simulink model "roach2_fengine"  (Simulink 8.1)
settings: Solver=VariableStepDiscrete  SolverMode=SingleTasking  StartTime=0.0  StopTime=10.0  RelTol=1e-3
undefined symbols (not defined in the file or in its folder): sfp
libraries: xbsIndex_r4 x3838, xps_library x64, casper_library_sources x16, casper_library_misc x13, casper_library_delays x7, casper_library_reorder x3
(part 4 of 43)

== system roach2_fengine/eq/7 ==
  note: Counter has length nchan=number of channels of the fft.
      | BRAM has length nchan as well. Every input/channel
      | has its own eq value.
      | Top half of the BRAM, has coefficients for first input,
      | second half for the rest.
  note: shared brams have a latency of three
  in : Inport
  sync : Inport  Port=2
  Constant4 : xbsIndex_r4/Constant  const=0  gui_display_data_type=Fixed-point  arith_type=Unsigned  n_bits=18  bin_pt=0  preci_type=Single  exp_width=8  frac_width=24  explicit_period=on  period=1  equ=P=C  opselect=C  inp2=PCIN>>17  opr=+  inp1=P  carry=CIN  dbl_ovrd=off  block_type=constant
  Constant5 : xbsIndex_r4/Constant  const=0  gui_display_data_type=Fixed-point  arith_type=Boolean  n_bits=1  bin_pt=0  preci_type=Single  exp_width=8  frac_width=24  explicit_period=on  period=1  equ=P=C  opselect=C  inp2=PCIN>>17  opr=+  inp1=P  carry=CIN  dbl_ovrd=off  block_type=constant
  Counter : xbsIndex_r4/Counter  cnt_type=Free Running  cnt_to=Inf  operation=Up  start_count=0  cnt_by_val=1  arith_type=Unsigned  n_bits=nchan  bin_pt=0  load_pin=off  rst=on  en=off  explicit_period=off  period=1  dbl_ovrd=off  use_behavioral_HDL=off  implementation=Fabric  use_rpm=on  block_type=counter
      settings: xl_area=[0, 0, 0, 0, 0, 0, 0]
  Delay1 : xbsIndex_r4/Delay  rst=off  infoeditControl=Selection of Reset will increase slice count due to use of real FFs and instead of SRLs  en=off  latency=4  dbl_ovrd=off  reg_retiming=on  block_type=delay
      settings: xl_area=[0, 0, 0, 0, 0, 0, 0]
  Delay11 : xbsIndex_r4/Delay  rst=off  infoeditControl=Selection of Reset will increase slice count due to use of real FFs and instead of SRLs  en=off  latency=4  dbl_ovrd=off  reg_retiming=on  block_type=delay
      settings: xl_area=[0, 0, 0, 0, 0, 0, 0]
  Delay2 : xbsIndex_r4/Delay  rst=off  infoeditControl=Selection of Reset will increase slice count due to use of real FFs and instead of SRLs  en=off  latency=3  dbl_ovrd=off  reg_retiming=off  block_type=delay
      settings: xl_area=[0, 0, 0, 0, 0, 0, 0]
  Delay3 : xbsIndex_r4/Delay  rst=off  infoeditControl=Selection of Reset will increase slice count due to use of real FFs and instead of SRLs  en=off  latency=4  dbl_ovrd=off  reg_retiming=on  block_type=delay
      settings: xl_area=[0, 0, 0, 0, 0, 0, 0]
  Delay4 : xbsIndex_r4/Delay  rst=off  infoeditControl=Selection of Reset will increase slice count due to use of real FFs and instead of SRLs  en=off  latency=5  dbl_ovrd=off  reg_retiming=off  block_type=delay
      settings: xl_area=[0, 0, 0, 0, 0, 0, 0]
  Delay5 : xbsIndex_r4/Delay  rst=off  infoeditControl=Selection of Reset will increase slice count due to use of real FFs and instead of SRLs  en=off  latency=3  dbl_ovrd=off  reg_retiming=off  block_type=delay
      settings: xl_area=[0, 0, 0, 0, 0, 0, 0]
  Slice1 : xbsIndex_r4/Slice  nbits=18  boolean_output=off  mode=Lower Bit Location + Width  bit1=0  base1=MSB of Input  bit0=0  base0=LSB of Input  dbl_ovrd=off  block_type=slice
      settings: block_version=10.1.3
  coeffs : Subsystem  Tag=xps:bram  AncestorBlock=xps_library/Shared BRAM  mask{arith_type=Unsigned, addr_width=nchan, data_width=32, reg_prim_output=on, reg_core_output=on, optimization=Minimum_Area, data_bin_pt=0, init_vals=[0:2^10-1], sample_rate=1}
      mask prompts: arith_type "Output Data Type"; addr_width "Address width"; data_width "Data Width"; reg_prim_output "Register Primitive Output"; reg_core_output "Register Core Output"; data_bin_pt "Data Binary Point"; init_vals "Initial 32-bit values (simulation only)"; sample_rate "Sample rate"
      mask init:
      | shared_bram_mask;
      mask TypeOptions arith_type: Unsigned | Signed  (2's comp)
      mask TypeOptions data_width: 8 | 16 | 32 | 64 | 128
      mask TypeOptions optimization: Minimum_Area | Low_Power
  round : Subsystem
      settings: AttributesFormatString=latency=5
  dout : Outport
  sync_out : Outport  Port=2
  clip : Outport  Port=3
  wires:
  Constant4/1 -> coeffs/2(data_in)
  Constant5/1 -> coeffs/3(we)
  sync/1 -> Counter/1, Delay5/1
  round/1(dout) -> dout/1
  in/1 -> Delay2/1
  Delay4/1 -> sync_out/1
  round/2(clip) -> clip/1
  coeffs/1(data_out) -> Slice1/1
  Counter/1 -> coeffs/1(addr)
  Delay1/1 -> round/2(scale)
  Slice1/1 -> Delay1/1
  Delay11/1 -> round/1(din)
  Delay2/1 -> Delay11/1
  Delay3/1 -> Delay4/1
  Delay5/1 -> Delay3/1

== system roach2_fengine/eq/8 ==
  note: shared brams have a latency of three
  note: Counter has length nchan=number of channels of the fft.
      | BRAM has length nchan as well. Every input/channel
      | has its own eq value.
      | Top half of the BRAM, has coefficients for first input,
      | second half for the rest.
  in : Inport
  sync : Inport  Port=2
  Constant4 : xbsIndex_r4/Constant  const=0  gui_display_data_type=Fixed-point  arith_type=Unsigned  n_bits=18  bin_pt=0  preci_type=Single  exp_width=8  frac_width=24  explicit_period=on  period=1  equ=P=C  opselect=C  inp2=PCIN>>17  opr=+  inp1=P  carry=CIN  dbl_ovrd=off  block_type=constant
  Constant5 : xbsIndex_r4/Constant  const=0  gui_display_data_type=Fixed-point  arith_type=Boolean  n_bits=1  bin_pt=0  preci_type=Single  exp_width=8  frac_width=24  explicit_period=on  period=1  equ=P=C  opselect=C  inp2=PCIN>>17  opr=+  inp1=P  carry=CIN  dbl_ovrd=off  block_type=constant
  Counter : xbsIndex_r4/Counter  cnt_type=Free Running  cnt_to=Inf  operation=Up  start_count=0  cnt_by_val=1  arith_type=Unsigned  n_bits=nchan  bin_pt=0  load_pin=off  rst=on  en=off  explicit_period=off  period=1  dbl_ovrd=off  use_behavioral_HDL=off  implementation=Fabric  use_rpm=on  block_type=counter
      settings: xl_area=[0, 0, 0, 0, 0, 0, 0]
  Delay1 : xbsIndex_r4/Delay  rst=off  infoeditControl=Selection of Reset will increase slice count due to use of real FFs and instead of SRLs  en=off  latency=4  dbl_ovrd=off  reg_retiming=on  block_type=delay
      settings: xl_area=[0, 0, 0, 0, 0, 0, 0]
  Delay11 : xbsIndex_r4/Delay  rst=off  infoeditControl=Selection of Reset will increase slice count due to use of real FFs and instead of SRLs  en=off  latency=4  dbl_ovrd=off  reg_retiming=on  block_type=delay
      settings: xl_area=[0, 0, 0, 0, 0, 0, 0]
  Delay2 : xbsIndex_r4/Delay  rst=off  infoeditControl=Selection of Reset will increase slice count due to use of real FFs and instead of SRLs  en=off  latency=3  dbl_ovrd=off  reg_retiming=off  block_type=delay
      settings: xl_area=[0, 0, 0, 0, 0, 0, 0]
  Delay3 : xbsIndex_r4/Delay  rst=off  infoeditControl=Selection of Reset will increase slice count due to use of real FFs and instead of SRLs  en=off  latency=4  dbl_ovrd=off  reg_retiming=on  block_type=delay
      settings: xl_area=[0, 0, 0, 0, 0, 0, 0]
  Delay4 : xbsIndex_r4/Delay  rst=off  infoeditControl=Selection of Reset will increase slice count due to use of real FFs and instead of SRLs  en=off  latency=5  dbl_ovrd=off  reg_retiming=off  block_type=delay
      settings: xl_area=[0, 0, 0, 0, 0, 0, 0]
  Delay5 : xbsIndex_r4/Delay  rst=off  infoeditControl=Selection of Reset will increase slice count due to use of real FFs and instead of SRLs  en=off  latency=3  dbl_ovrd=off  reg_retiming=off  block_type=delay
      settings: xl_area=[0, 0, 0, 0, 0, 0, 0]
  Slice1 : xbsIndex_r4/Slice  nbits=18  boolean_output=off  mode=Lower Bit Location + Width  bit1=0  base1=MSB of Input  bit0=0  base0=LSB of Input  dbl_ovrd=off  block_type=slice
      settings: block_version=10.1.3
  coeffs : Subsystem  Tag=xps:bram  AncestorBlock=xps_library/Shared BRAM  mask{arith_type=Unsigned, addr_width=nchan, data_width=32, reg_prim_output=on, reg_core_output=on, optimization=Minimum_Area, data_bin_pt=0, init_vals=[0:2^10-1], sample_rate=1}
      mask prompts: arith_type "Output Data Type"; addr_width "Address width"; data_width "Data Width"; reg_prim_output "Register Primitive Output"; reg_core_output "Register Core Output"; data_bin_pt "Data Binary Point"; init_vals "Initial 32-bit values (simulation only)"; sample_rate "Sample rate"
      mask init:
      | shared_bram_mask;
      mask TypeOptions arith_type: Unsigned | Signed  (2's comp)
      mask TypeOptions data_width: 8 | 16 | 32 | 64 | 128
      mask TypeOptions optimization: Minimum_Area | Low_Power
  round : Subsystem
      settings: AttributesFormatString=latency=5
  dout : Outport
  sync_out : Outport  Port=2
  clip : Outport  Port=3
  wires:
  Delay5/1 -> Delay3/1
  Delay3/1 -> Delay4/1
  Delay2/1 -> Delay11/1
  Delay11/1 -> round/1(din)
  Slice1/1 -> Delay1/1
  Delay1/1 -> round/2(scale)
  Counter/1 -> coeffs/1(addr)
  coeffs/1(data_out) -> Slice1/1
  round/2(clip) -> clip/1
  Delay4/1 -> sync_out/1
  in/1 -> Delay2/1
  round/1(dout) -> dout/1
  sync/1 -> Delay5/1, Counter/1
  Constant5/1 -> coeffs/3(we)
  Constant4/1 -> coeffs/2(data_in)

== system roach2_fengine/eq/9 ==
  note: shared brams have a latency of three
  note: Counter has length nchan=number of channels of the fft.
      | BRAM has length nchan as well. Every input/channel
      | has its own eq value.
      | Top half of the BRAM, has coefficients for first input,
      | second half for the rest.
  in : Inport
  sync : Inport  Port=2
  Constant4 : xbsIndex_r4/Constant  const=0  gui_display_data_type=Fixed-point  arith_type=Unsigned  n_bits=18  bin_pt=0  preci_type=Single  exp_width=8  frac_width=24  explicit_period=on  period=1  equ=P=C  opselect=C  inp2=PCIN>>17  opr=+  inp1=P  carry=CIN  dbl_ovrd=off  block_type=constant
  Constant5 : xbsIndex_r4/Constant  const=0  gui_display_data_type=Fixed-point  arith_type=Boolean  n_bits=1  bin_pt=0  preci_type=Single  exp_width=8  frac_width=24  explicit_period=on  period=1  equ=P=C  opselect=C  inp2=PCIN>>17  opr=+  inp1=P  carry=CIN  dbl_ovrd=off  block_type=constant
  Counter : xbsIndex_r4/Counter  cnt_type=Free Running  cnt_to=Inf  operation=Up  start_count=0  cnt_by_val=1  arith_type=Unsigned  n_bits=nchan  bin_pt=0  load_pin=off  rst=on  en=off  explicit_period=off  period=1  dbl_ovrd=off  use_behavioral_HDL=off  implementation=Fabric  use_rpm=on  block_type=counter
      settings: xl_area=[0, 0, 0, 0, 0, 0, 0]
  Delay1 : xbsIndex_r4/Delay  rst=off  infoeditControl=Selection of Reset will increase slice count due to use of real FFs and instead of SRLs  en=off  latency=4  dbl_ovrd=off  reg_retiming=on  block_type=delay
      settings: xl_area=[0, 0, 0, 0, 0, 0, 0]
  Delay11 : xbsIndex_r4/Delay  rst=off  infoeditControl=Selection of Reset will increase slice count due to use of real FFs and instead of SRLs  en=off  latency=4  dbl_ovrd=off  reg_retiming=on  block_type=delay
      settings: xl_area=[0, 0, 0, 0, 0, 0, 0]
  Delay2 : xbsIndex_r4/Delay  rst=off  infoeditControl=Selection of Reset will increase slice count due to use of real FFs and instead of SRLs  en=off  latency=3  dbl_ovrd=off  reg_retiming=off  block_type=delay
      settings: xl_area=[0, 0, 0, 0, 0, 0, 0]
  Delay3 : xbsIndex_r4/Delay  rst=off  infoeditControl=Selection of Reset will increase slice count due to use of real FFs and instead of SRLs  en=off  latency=4  dbl_ovrd=off  reg_retiming=on  block_type=delay
      settings: xl_area=[0, 0, 0, 0, 0, 0, 0]
  Delay4 : xbsIndex_r4/Delay  rst=off  infoeditControl=Selection of Reset will increase slice count due to use of real FFs and instead of SRLs  en=off  latency=5  dbl_ovrd=off  reg_retiming=off  block_type=delay
      settings: xl_area=[0, 0, 0, 0, 0, 0, 0]
  Delay5 : xbsIndex_r4/Delay  rst=off  infoeditControl=Selection of Reset will increase slice count due to use of real FFs and instead of SRLs  en=off  latency=3  dbl_ovrd=off  reg_retiming=off  block_type=delay
      settings: xl_area=[0, 0, 0, 0, 0, 0, 0]
  Slice1 : xbsIndex_r4/Slice  nbits=18  boolean_output=off  mode=Lower Bit Location + Width  bit1=0  base1=MSB of Input  bit0=0  base0=LSB of Input  dbl_ovrd=off  block_type=slice
      settings: block_version=10.1.3
  coeffs : Subsystem  Tag=xps:bram  AncestorBlock=xps_library/Shared BRAM  mask{arith_type=Unsigned, addr_width=nchan, data_width=32, reg_prim_output=on, reg_core_output=on, optimization=Minimum_Area, data_bin_pt=0, init_vals=[0:2^10-1], sample_rate=1}
      mask prompts: arith_type "Output Data Type"; addr_width "Address width"; data_width "Data Width"; reg_prim_output "Register Primitive Output"; reg_core_output "Register Core Output"; data_bin_pt "Data Binary Point"; init_vals "Initial 32-bit values (simulation only)"; sample_rate "Sample rate"
      mask init:
      | shared_bram_mask;
      mask TypeOptions arith_type: Unsigned | Signed  (2's comp)
      mask TypeOptions data_width: 8 | 16 | 32 | 64 | 128
      mask TypeOptions optimization: Minimum_Area | Low_Power
  round : Subsystem
      settings: AttributesFormatString=latency=5
  dout : Outport
  sync_out : Outport  Port=2
  clip : Outport  Port=3
  wires:
  Delay5/1 -> Delay3/1
  Delay3/1 -> Delay4/1
  Delay2/1 -> Delay11/1
  Delay11/1 -> round/1(din)
  Slice1/1 -> Delay1/1
  Delay1/1 -> round/2(scale)
  Counter/1 -> coeffs/1(addr)
  coeffs/1(data_out) -> Slice1/1
  round/2(clip) -> clip/1
  Delay4/1 -> sync_out/1
  in/1 -> Delay2/1
  round/1(dout) -> dout/1
  sync/1 -> Delay5/1, Counter/1
  Constant5/1 -> coeffs/3(we)
  Constant4/1 -> coeffs/2(data_in)

== system roach2_fengine/eq/eq_snap ==
  In1 : Inport
  In2 : Inport  Port=2
  In3 : Inport  Port=3
  In4 : Inport  Port=4
  BitBasher : xbsIndex_r4/BitBasher  bitexpr=|  display_expr=off  sr_1=1  arith_type1=Unsigned  bin_pt1=0  sr_2=2  arith_type2=Unsigned  bin_pt2=0  sr_3=3  arith_type3=Unsigned  bin_pt3=0  sr_4=4  arith_type4=Unsigned  bin_pt4=0  dbl_ovrd=off  block_type=bitbasher
      | <!DOCTYPE HTML PUBLIC "-//W3C//DTD HTML 4.0//EN" "http://www.w3.org/TR/REC-html40/strict.dtd">
      | <html><head><meta name="qrichtext" content="1" /><style type="text/css">
      | p, li { white-space: pre-wrap; }
      | </style></head><body style=" font-family:'Sans'; font-size:10pt; font-weight:400; font-style:normal;">
      | <p style=" margin-top:12px; margin-bottom:12px; margin-left:0px; margin-right:0px; -qt-block-indent:0; text-indent:0px;"><span style=" font-family:'MS Shell Dlg'; font-size:8pt;">w32={d8,c8,b8,a8}</span></p></body></html>
  Delay : xbsIndex_r4/Delay  rst=off  infoeditControl=Selection of Reset will increase slice count due to use of real FFs and instead of SRLs  en=off  latency=3  dbl_ovrd=off  reg_retiming=on  block_type=delay
  tag scope: eq_snap=global
  wires:
  BitBasher/1 -> Delay/1
  Delay/1 -> [eq_snap]
  In1/1 -> BitBasher/1
  In2/1 -> BitBasher/2
  In3/1 -> BitBasher/3
  In4/1 -> BitBasher/4

== system roach2_fengine/eth/0 ==
  data : Inport
  Bus Selector : BusSelector  OutputSignals=dout,valid
  Bus Selector1 : BusSelector  OutputSignals=eof
  Bus Selector3 : BusSelector  OutputSignals=dest
  Concat : xbsIndex_r4/Concat  num_inputs=8  dbl_ovrd=off  block_type=concat
  Constant : xbsIndex_r4/Constant  const=8511  gui_display_data_type=Fixed-point  arith_type=Unsigned  n_bits=16  bin_pt=0  preci_type=Single  exp_width=8  frac_width=24  explicit_period=on  period=1  equ=P=C  opselect=C  inp2=PCIN>>17  opr=+  inp1=P  carry=CIN  dbl_ovrd=off  block_type=constant
  Constant3 : xbsIndex_r4/Constant  const=0  gui_display_data_type=Fixed-point  arith_type=Unsigned  n_bits=25  bin_pt=0  preci_type=Single  exp_width=8  frac_width=24  explicit_period=on  period=1  equ=P=C  opselect=C  inp2=PCIN>>17  opr=+  inp1=P  carry=CIN  dbl_ovrd=off  block_type=constant
      settings: block_version=11.4
  Delay3 : xbsIndex_r4/Delay  rst=off  infoeditControl=Selection of Reset will increase slice count due to use of real FFs and instead of SRLs  en=off  latency=1  dbl_ovrd=off  reg_retiming=off  block_type=delay
  Terminator{4..9} (6 alike) : Terminator
  gbe_sw_en : Subsystem
  sw : xps_library/ten_Gbe_v2  Tag=xps:tengbe_v2  flavour=sfp+  slot=0  port_r1=0  port_r2_cx4=0  port_r2_sfpp=0  rx_dist_ram=on  large_frames=on  show_param=on  pre_emph_r2=0.74  pre_emph=3  post_emph_r2=0.18  rxeqmix_r2=7  swing_r2=940  swing=800  fab_en=on  fab_mac=hex2dec('02020a0a0a20')  fab_ip=hex2dec('0a0a0a20')  fab_udp=8511  fab_gate=1  cpu_rx_en=off  cpu_tx_en=off
  sw_bframes : xps_library/software register  Tag=xps:sw_reg  io_dir=To Processor  arith_type=Unsigned  bitwidth=32  bin_pt=0  sample_period=1  gw_name=roach2_fengine_eth_0_sw_bframes_user_data_in
  sw_mon : Subsystem
  sw_oflows : xps_library/software register  Tag=xps:sw_reg  io_dir=To Processor  arith_type=Unsigned  bitwidth=32  bin_pt=0  sample_period=1  gw_name=roach2_fengine_eth_0_sw_oflows_user_data_in
  sw_oruns : xps_library/software register  Tag=xps:sw_reg  io_dir=To Processor  arith_type=Unsigned  bitwidth=32  bin_pt=0  sample_period=1  gw_name=roach2_fengine_eth_0_sw_oruns_user_data_in
  sw_status : xps_library/software register  Tag=xps:sw_reg  io_dir=To Processor  arith_type=Unsigned  bitwidth=32  bin_pt=0  sample_period=1  gw_name=roach2_fengine_eth_0_sw_status_user_data_in
  wires:
  sw_status/1 -> Terminator9/1
  sw_oruns/1 -> Terminator8/1
  sw_oflows/1 -> Terminator7/1
  sw_bframes/1 -> Terminator4/1
  Bus Selector/1 -> sw/2  "<dout>"
  Bus Selector/2 -> gbe_sw_en/1(in)  "<valid>"
  data/1 -> Bus Selector3/1, Bus Selector/1, Bus Selector1/1
  Bus Selector1/1 -> sw/6  "<eof>"
  Constant/1 -> sw/5
  sw/7 -> sw/7
  sw/12 -> sw/8, [gbe_sw_rx_orun]
  [gbe_sw_rst] -> sw/1
  gbe_sw_en/1(out) -> sw/3
  sw/1 -> [gbe_sw_led_up]
  sw/2 -> [gbe_sw_led_rx]
  sw/3 -> [gbe_sw_led_tx]
  sw/4 -> [gbe_sw_tx_afull]
  sw/5 -> [gbe_sw_tx_oflow]
  sw/8 -> Terminator5/1
  sw/9 -> Terminator6/1
  sw/11 -> [gbe_sw_rx_bfr]
  [gbe_sw_rx_bfr] -> sw_mon/1(bfr)
  [gbe_sw_rx_orun] -> sw_mon/2(orun)
  [gbe_sw_tx_oflow] -> sw_mon/3(oflow)
  Concat/1 -> Delay3/1
  Constant3/1 -> Concat/1
  [gbe_sw_led_up] -> Concat/2
  [gbe_sw_led_rx] -> Concat/3
  [gbe_sw_led_tx] -> Concat/4
  [gbe_sw_tx_afull] -> Concat/5
  [gbe_sw_tx_oflow] -> Concat/6
  [gbe_sw_rx_bfr] -> Concat/7
  [gbe_sw_rx_orun] -> Concat/8
  Bus Selector3/1 -> sw/4  "<dest>"
  Delay3/1 -> sw_status/1
  sw_mon/1(bfr_cnt) -> sw_bframes/1
  sw_mon/3(oflow_cnt) -> sw_oflows/1
  sw_mon/2(orun_cnt) -> sw_oruns/1

== system roach2_fengine/eth/1 ==
  data : Inport
  Bus Selector : BusSelector  OutputSignals=dout,valid
  Bus Selector1 : BusSelector  OutputSignals=eof
  Bus Selector3 : BusSelector  OutputSignals=dest
  Concat : xbsIndex_r4/Concat  num_inputs=8  dbl_ovrd=off  block_type=concat
  Constant : xbsIndex_r4/Constant  const=8511  gui_display_data_type=Fixed-point  arith_type=Unsigned  n_bits=16  bin_pt=0  preci_type=Single  exp_width=8  frac_width=24  explicit_period=on  period=1  equ=P=C  opselect=C  inp2=PCIN>>17  opr=+  inp1=P  carry=CIN  dbl_ovrd=off  block_type=constant
  Constant3 : xbsIndex_r4/Constant  const=0  gui_display_data_type=Fixed-point  arith_type=Unsigned  n_bits=25  bin_pt=0  preci_type=Single  exp_width=8  frac_width=24  explicit_period=on  period=1  equ=P=C  opselect=C  inp2=PCIN>>17  opr=+  inp1=P  carry=CIN  dbl_ovrd=off  block_type=constant
      settings: block_version=11.4
  Delay3 : xbsIndex_r4/Delay  rst=off  infoeditControl=Selection of Reset will increase slice count due to use of real FFs and instead of SRLs  en=off  latency=1  dbl_ovrd=off  reg_retiming=off  block_type=delay
  Terminator{4..9} (6 alike) : Terminator
  gbe_sw_en : Subsystem
  sw : xps_library/ten_Gbe_v2  Tag=xps:tengbe_v2  flavour=sfp+  slot=0  port_r1=0  port_r2_cx4=0  port_r2_sfpp=1  rx_dist_ram=on  large_frames=on  show_param=on  pre_emph_r2=0.74  pre_emph=3  post_emph_r2=0.18  rxeqmix_r2=7  swing_r2=940  swing=800  fab_en=on  fab_mac=hex2dec('02020a0a0a20')  fab_ip=hex2dec('0a0a0a20')  fab_udp=8511  fab_gate=1  cpu_rx_en=off  cpu_tx_en=off
  sw_bframes : xps_library/software register  Tag=xps:sw_reg  io_dir=To Processor  arith_type=Unsigned  bitwidth=32  bin_pt=0  sample_period=1  gw_name=roach2_fengine_eth_1_sw_bframes_user_data_in
  sw_mon : Subsystem
  sw_oflows : xps_library/software register  Tag=xps:sw_reg  io_dir=To Processor  arith_type=Unsigned  bitwidth=32  bin_pt=0  sample_period=1  gw_name=roach2_fengine_eth_1_sw_oflows_user_data_in
  sw_oruns : xps_library/software register  Tag=xps:sw_reg  io_dir=To Processor  arith_type=Unsigned  bitwidth=32  bin_pt=0  sample_period=1  gw_name=roach2_fengine_eth_1_sw_oruns_user_data_in
  sw_status : xps_library/software register  Tag=xps:sw_reg  io_dir=To Processor  arith_type=Unsigned  bitwidth=32  bin_pt=0  sample_period=1  gw_name=roach2_fengine_eth_1_sw_status_user_data_in
  wires:
  sw_mon/2(orun_cnt) -> sw_oruns/1
  sw_mon/3(oflow_cnt) -> sw_oflows/1
  sw_mon/1(bfr_cnt) -> sw_bframes/1
  Delay3/1 -> sw_status/1
  Bus Selector3/1 -> sw/4  "<dest>"
  [gbe_sw_rx_orun] -> Concat/8
  [gbe_sw_rx_bfr] -> Concat/7
  [gbe_sw_tx_oflow] -> Concat/6
  [gbe_sw_tx_afull] -> Concat/5
  [gbe_sw_led_tx] -> Concat/4
  [gbe_sw_led_rx] -> Concat/3
  [gbe_sw_led_up] -> Concat/2
  Constant3/1 -> Concat/1
  Concat/1 -> Delay3/1
  [gbe_sw_tx_oflow] -> sw_mon/3(oflow)
  [gbe_sw_rx_orun] -> sw_mon/2(orun)
  [gbe_sw_rx_bfr] -> sw_mon/1(bfr)
  sw/11 -> [gbe_sw_rx_bfr]
  sw/9 -> Terminator6/1
  sw/8 -> Terminator5/1
  sw/5 -> [gbe_sw_tx_oflow]
  sw/4 -> [gbe_sw_tx_afull]
  sw/3 -> [gbe_sw_led_tx]
  sw/2 -> [gbe_sw_led_rx]
  sw/1 -> [gbe_sw_led_up]
  gbe_sw_en/1(out) -> sw/3
  [gbe_sw_rst] -> sw/1
  sw/12 -> [gbe_sw_rx_orun], sw/8
  sw/7 -> sw/7
  Constant/1 -> sw/5
  Bus Selector1/1 -> sw/6  "<eof>"
  data/1 -> Bus Selector1/1, Bus Selector/1, Bus Selector3/1
  Bus Selector/2 -> gbe_sw_en/1(in)  "<valid>"
  Bus Selector/1 -> sw/2  "<dout>"
  sw_bframes/1 -> Terminator4/1
  sw_oflows/1 -> Terminator7/1
  sw_oruns/1 -> Terminator8/1
  sw_status/1 -> Terminator9/1

== system roach2_fengine/eth/2 ==
  data : Inport
  Bus Selector : BusSelector  OutputSignals=dout,valid
  Bus Selector1 : BusSelector  OutputSignals=eof
  Bus Selector3 : BusSelector  OutputSignals=dest
  Concat : xbsIndex_r4/Concat  num_inputs=8  dbl_ovrd=off  block_type=concat
  Constant : xbsIndex_r4/Constant  const=8511  gui_display_data_type=Fixed-point  arith_type=Unsigned  n_bits=16  bin_pt=0  preci_type=Single  exp_width=8  frac_width=24  explicit_period=on  period=1  equ=P=C  opselect=C  inp2=PCIN>>17  opr=+  inp1=P  carry=CIN  dbl_ovrd=off  block_type=constant
  Constant3 : xbsIndex_r4/Constant  const=0  gui_display_data_type=Fixed-point  arith_type=Unsigned  n_bits=25  bin_pt=0  preci_type=Single  exp_width=8  frac_width=24  explicit_period=on  period=1  equ=P=C  opselect=C  inp2=PCIN>>17  opr=+  inp1=P  carry=CIN  dbl_ovrd=off  block_type=constant
      settings: block_version=11.4
  Delay3 : xbsIndex_r4/Delay  rst=off  infoeditControl=Selection of Reset will increase slice count due to use of real FFs and instead of SRLs  en=off  latency=1  dbl_ovrd=off  reg_retiming=off  block_type=delay
  Terminator{4..9} (6 alike) : Terminator
  gbe_sw_en : Subsystem
  sw : xps_library/ten_Gbe_v2  Tag=xps:tengbe_v2  flavour=sfp+  slot=0  port_r1=0  port_r2_cx4=0  port_r2_sfpp=2  rx_dist_ram=on  large_frames=on  show_param=on  pre_emph_r2=0.74  pre_emph=3  post_emph_r2=0.18  rxeqmix_r2=7  swing_r2=940  swing=800  fab_en=on  fab_mac=hex2dec('02020a0a0a20')  fab_ip=hex2dec('0a0a0a20')  fab_udp=8511  fab_gate=1  cpu_rx_en=off  cpu_tx_en=off
  sw_bframes : xps_library/software register  Tag=xps:sw_reg  io_dir=To Processor  arith_type=Unsigned  bitwidth=32  bin_pt=0  sample_period=1  gw_name=roach2_fengine_eth_2_sw_bframes_user_data_in
  sw_mon : Subsystem
  sw_oflows : xps_library/software register  Tag=xps:sw_reg  io_dir=To Processor  arith_type=Unsigned  bitwidth=32  bin_pt=0  sample_period=1  gw_name=roach2_fengine_eth_2_sw_oflows_user_data_in
  sw_oruns : xps_library/software register  Tag=xps:sw_reg  io_dir=To Processor  arith_type=Unsigned  bitwidth=32  bin_pt=0  sample_period=1  gw_name=roach2_fengine_eth_2_sw_oruns_user_data_in
  sw_status : xps_library/software register  Tag=xps:sw_reg  io_dir=To Processor  arith_type=Unsigned  bitwidth=32  bin_pt=0  sample_period=1  gw_name=roach2_fengine_eth_2_sw_status_user_data_in
  wires:
  sw_mon/2(orun_cnt) -> sw_oruns/1
  sw_mon/3(oflow_cnt) -> sw_oflows/1
  sw_mon/1(bfr_cnt) -> sw_bframes/1
  Delay3/1 -> sw_status/1
  Bus Selector3/1 -> sw/4  "<dest>"
  [gbe_sw_rx_orun] -> Concat/8
  [gbe_sw_rx_bfr] -> Concat/7
  [gbe_sw_tx_oflow] -> Concat/6
  [gbe_sw_tx_afull] -> Concat/5
  [gbe_sw_led_tx] -> Concat/4
  [gbe_sw_led_rx] -> Concat/3
  [gbe_sw_led_up] -> Concat/2
  Constant3/1 -> Concat/1
  Concat/1 -> Delay3/1
  [gbe_sw_tx_oflow] -> sw_mon/3(oflow)
  [gbe_sw_rx_orun] -> sw_mon/2(orun)
  [gbe_sw_rx_bfr] -> sw_mon/1(bfr)
  sw/11 -> [gbe_sw_rx_bfr]
  sw/9 -> Terminator6/1
  sw/8 -> Terminator5/1
  sw/5 -> [gbe_sw_tx_oflow]
  sw/4 -> [gbe_sw_tx_afull]
  sw/3 -> [gbe_sw_led_tx]
  sw/2 -> [gbe_sw_led_rx]
  sw/1 -> [gbe_sw_led_up]
  gbe_sw_en/1(out) -> sw/3
  [gbe_sw_rst] -> sw/1
  sw/12 -> [gbe_sw_rx_orun], sw/8
  sw/7 -> sw/7
  Constant/1 -> sw/5
  Bus Selector1/1 -> sw/6  "<eof>"
  data/1 -> Bus Selector1/1, Bus Selector/1, Bus Selector3/1
  Bus Selector/2 -> gbe_sw_en/1(in)  "<valid>"
  Bus Selector/1 -> sw/2  "<dout>"
  sw_bframes/1 -> Terminator4/1
  sw_oflows/1 -> Terminator7/1
  sw_oruns/1 -> Terminator8/1
  sw_status/1 -> Terminator9/1

== system roach2_fengine/eth/3 ==
  data : Inport
  Bus Selector : BusSelector  OutputSignals=dout,valid
  Bus Selector1 : BusSelector  OutputSignals=eof
  Bus Selector3 : BusSelector  OutputSignals=dest
  Concat : xbsIndex_r4/Concat  num_inputs=8  dbl_ovrd=off  block_type=concat
  Constant : xbsIndex_r4/Constant  const=8511  gui_display_data_type=Fixed-point  arith_type=Unsigned  n_bits=16  bin_pt=0  preci_type=Single  exp_width=8  frac_width=24  explicit_period=on  period=1  equ=P=C  opselect=C  inp2=PCIN>>17  opr=+  inp1=P  carry=CIN  dbl_ovrd=off  block_type=constant
  Constant3 : xbsIndex_r4/Constant  const=0  gui_display_data_type=Fixed-point  arith_type=Unsigned  n_bits=25  bin_pt=0  preci_type=Single  exp_width=8  frac_width=24  explicit_period=on  period=1  equ=P=C  opselect=C  inp2=PCIN>>17  opr=+  inp1=P  carry=CIN  dbl_ovrd=off  block_type=constant
      settings: block_version=11.4
  Delay3 : xbsIndex_r4/Delay  rst=off  infoeditControl=Selection of Reset will increase slice count due to use of real FFs and instead of SRLs  en=off  latency=1  dbl_ovrd=off  reg_retiming=off  block_type=delay
  Terminator{4..9} (6 alike) : Terminator
  gbe_sw_en : Subsystem
  sw : xps_library/ten_Gbe_v2  Tag=xps:tengbe_v2  flavour=sfp+  slot=0  port_r1=0  port_r2_cx4=0  port_r2_sfpp=3  rx_dist_ram=on  large_frames=on  show_param=on  pre_emph_r2=0.74  pre_emph=3  post_emph_r2=0.18  rxeqmix_r2=7  swing_r2=940  swing=800  fab_en=on  fab_mac=hex2dec('02020a0a0a20')  fab_ip=hex2dec('0a0a0a20')  fab_udp=8511  fab_gate=1  cpu_rx_en=off  cpu_tx_en=off
  sw_bframes : xps_library/software register  Tag=xps:sw_reg  io_dir=To Processor  arith_type=Unsigned  bitwidth=32  bin_pt=0  sample_period=1  gw_name=roach2_fengine_eth_3_sw_bframes_user_data_in
  sw_mon : Subsystem
  sw_oflows : xps_library/software register  Tag=xps:sw_reg  io_dir=To Processor  arith_type=Unsigned  bitwidth=32  bin_pt=0  sample_period=1  gw_name=roach2_fengine_eth_3_sw_oflows_user_data_in
  sw_oruns : xps_library/software register  Tag=xps:sw_reg  io_dir=To Processor  arith_type=Unsigned  bitwidth=32  bin_pt=0  sample_period=1  gw_name=roach2_fengine_eth_3_sw_oruns_user_data_in
  sw_status : xps_library/software register  Tag=xps:sw_reg  io_dir=To Processor  arith_type=Unsigned  bitwidth=32  bin_pt=0  sample_period=1  gw_name=roach2_fengine_eth_3_sw_status_user_data_in
  wires:
  sw_status/1 -> Terminator9/1
  sw_oruns/1 -> Terminator8/1
  sw_oflows/1 -> Terminator7/1
  sw_bframes/1 -> Terminator4/1
  Bus Selector/1 -> sw/2  "<dout>"
  Bus Selector/2 -> gbe_sw_en/1(in)  "<valid>"
  data/1 -> Bus Selector3/1, Bus Selector/1, Bus Selector1/1
  Bus Selector1/1 -> sw/6  "<eof>"
  Constant/1 -> sw/5
  sw/7 -> sw/7
  sw/12 -> sw/8, [gbe_sw_rx_orun]
  [gbe_sw_rst] -> sw/1
  gbe_sw_en/1(out) -> sw/3
  sw/1 -> [gbe_sw_led_up]
  sw/2 -> [gbe_sw_led_rx]
  sw/3 -> [gbe_sw_led_tx]
  sw/4 -> [gbe_sw_tx_afull]
  sw/5 -> [gbe_sw_tx_oflow]
  sw/8 -> Terminator5/1
  sw/9 -> Terminator6/1
  sw/11 -> [gbe_sw_rx_bfr]
  [gbe_sw_rx_bfr] -> sw_mon/1(bfr)
  [gbe_sw_rx_orun] -> sw_mon/2(orun)
  [gbe_sw_tx_oflow] -> sw_mon/3(oflow)
  Concat/1 -> Delay3/1
  Constant3/1 -> Concat/1
  [gbe_sw_led_up] -> Concat/2
  [gbe_sw_led_rx] -> Concat/3
  [gbe_sw_led_tx] -> Concat/4
  [gbe_sw_tx_afull] -> Concat/5
  [gbe_sw_tx_oflow] -> Concat/6
  [gbe_sw_rx_bfr] -> Concat/7
  [gbe_sw_rx_orun] -> Concat/8
  Bus Selector3/1 -> sw/4  "<dest>"
  Delay3/1 -> sw_status/1
  sw_mon/1(bfr_cnt) -> sw_bframes/1
  sw_mon/3(oflow_cnt) -> sw_oflows/1
  sw_mon/2(orun_cnt) -> sw_oruns/1

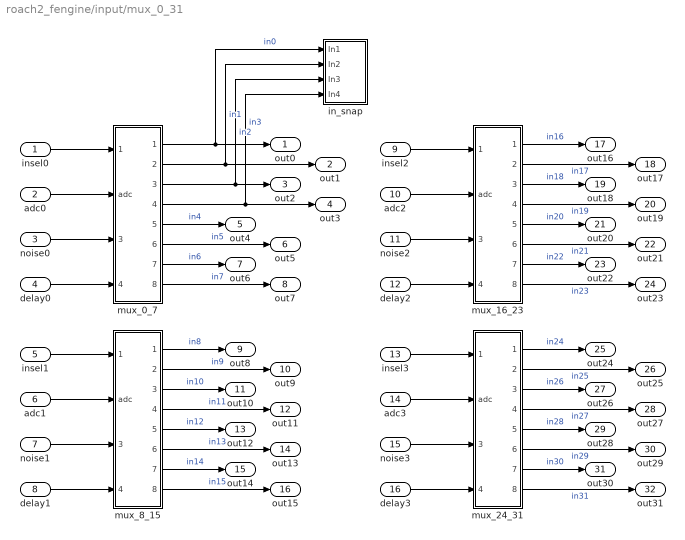
== system roach2_fengine/input/mux_0_31 ==
  insel0 : Inport
  adc0 : Inport  Port=2
  noise0 : Inport  Port=3
  delay0 : Inport  Port=4
  insel1 : Inport  Port=5
  adc1 : Inport  Port=6
  noise1 : Inport  Port=7
  delay1 : Inport  Port=8
  insel2 : Inport  Port=9
  adc2 : Inport  Port=10
  noise2 : Inport  Port=11
  delay2 : Inport  Port=12
  insel3 : Inport  Port=13
  adc3 : Inport  Port=14
  noise3 : Inport  Port=15
  delay3 : Inport  Port=16
  in_snap : Subsystem
  mux_0_7 : Subsystem
  mux_16_23 : Subsystem
  mux_24_31 : Subsystem
  mux_8_15 : Subsystem
  out0 : Outport
  out1 : Outport  Port=2
  out2 : Outport  Port=3
  out3 : Outport  Port=4
  out4 : Outport  Port=5
  out5 : Outport  Port=6
  out6 : Outport  Port=7
  out7 : Outport  Port=8
  out8 : Outport  Port=9
  out9 : Outport  Port=10
  out10 : Outport  Port=11
  out11 : Outport  Port=12
  out12 : Outport  Port=13
  out13 : Outport  Port=14
  out14 : Outport  Port=15
  out15 : Outport  Port=16
  out16 : Outport  Port=17
  out17 : Outport  Port=18
  out18 : Outport  Port=19
  out19 : Outport  Port=20
  out20 : Outport  Port=21
  out21 : Outport  Port=22
  out22 : Outport  Port=23
  out23 : Outport  Port=24
  out24 : Outport  Port=25
  out25 : Outport  Port=26
  out26 : Outport  Port=27
  out27 : Outport  Port=28
  out28 : Outport  Port=29
  out29 : Outport  Port=30
  out30 : Outport  Port=31
  out31 : Outport  Port=32
  wires:
  insel0/1 -> mux_0_7/1(insel)
  mux_0_7/1(out0) -> out0/1, in_snap/1(In1)  "in0"
  mux_0_7/2(out1) -> out1/1, in_snap/2(In2)  "in1"
  mux_0_7/3(out2) -> out2/1, in_snap/3(In3)  "in2"
  adc0/1 -> mux_0_7/2(adc)
  mux_0_7/4(out3) -> out3/1, in_snap/4(In4)  "in3"
  mux_0_7/5(out4) -> out4/1  "in4"
  noise0/1 -> mux_0_7/3(noise)
  mux_0_7/6(out5) -> out5/1  "in5"
  mux_0_7/7(out6) -> out6/1  "in6"
  delay0/1 -> mux_0_7/4(delay)
  mux_0_7/8(out7) -> out7/1  "in7"
  mux_8_15/1(out0) -> out8/1  "in8"
  insel1/1 -> mux_8_15/1(insel)
  mux_8_15/2(out1) -> out9/1  "in9"
  mux_8_15/3(out2) -> out10/1  "in10"
  adc1/1 -> mux_8_15/2(adc)
  mux_8_15/4(out3) -> out11/1  "in11"
  mux_8_15/5(out4) -> out12/1  "in12"
  noise1/1 -> mux_8_15/3(noise)
  mux_8_15/6(out5) -> out13/1  "in13"
  mux_8_15/7(out6) -> out14/1  "in14"
  delay1/1 -> mux_8_15/4(delay)
  mux_8_15/8(out7) -> out15/1  "in15"
  mux_16_23/1(out0) -> out16/1  "in16"
  insel2/1 -> mux_16_23/1(insel)
  mux_16_23/2(out1) -> out17/1  "in17"
  mux_16_23/3(out2) -> out18/1  "in18"
  adc2/1 -> mux_16_23/2(adc)
  mux_16_23/4(out3) -> out19/1  "in19"
  mux_16_23/5(out4) -> out20/1  "in20"
  noise2/1 -> mux_16_23/3(noise)
  mux_16_23/6(out5) -> out21/1  "in21"
  mux_16_23/7(out6) -> out22/1  "in22"
  delay2/1 -> mux_16_23/4(delay)
  mux_16_23/8(out7) -> out23/1  "in23"
  mux_24_31/1(out0) -> out24/1  "in24"
  insel3/1 -> mux_24_31/1(insel)
  mux_24_31/2(out1) -> out25/1  "in25"
  mux_24_31/3(out2) -> out26/1  "in26"
  adc3/1 -> mux_24_31/2(adc)
  mux_24_31/4(out3) -> out27/1  "in27"
  mux_24_31/5(out4) -> out28/1  "in28"
  noise3/1 -> mux_24_31/3(noise)
  mux_24_31/6(out5) -> out29/1  "in29"
  mux_24_31/7(out6) -> out30/1  "in30"
  delay3/1 -> mux_24_31/4(delay)
  mux_24_31/8(out7) -> out31/1  "in31"

== system roach2_fengine/input/rms ==
  note: To provide a means for measuring the magnitude of the signals
      | coming in to each ADC channel, we have a "calc_adc_sum_sq"
      | block which uses a single multiplier, two accumulators, and two
      | regsiters to calculate the sum of 64K ADC samples and the sum
      | of squares of 64K ADC samples.  These can be used to calculate
      | the mean (aka DC offset) and standard deviation (aka RMS) of
      | the ADC inputs.
  note: The sum_sumsq values are Fix_56_0.
      | The upper 24 bits are Fix_24_0 sum of samples.
      | The lower 32 bits are UFix_32_0 sum of squares.
  note: sum,sq,sum,sq,...
  in : Inport
  Bus Selector : BusSelector  OutputSignals=in0,in1,in2,in3,in4,in5,in6,in7,in8,in9,in10,in11,in12,in13,in14,in15,in16,in17,in18,in19,in20,in21,in22,in23,in24,in25,in26,in27,in28,in29,in30,in31
  Constant : Constant  Value=0
  Counter : xbsIndex_r4/Counter  cnt_type=Free Running  cnt_to=Inf  operation=Up  start_count=0  cnt_by_val=1  arith_type=Unsigned  n_bits=16  bin_pt=0  load_pin=off  rst=off  en=off  explicit_period=off  period=1  dbl_ovrd=off  use_behavioral_HDL=off  implementation=Fabric  use_rpm=on  block_type=counter
      settings: xl_area=[0, 0, 0, 0, 0, 0, 0]
  Slice16 : xbsIndex_r4/Slice  nbits=1  boolean_output=on  mode=Upper Bit Location + Width  bit1=0  base1=MSB of Input  bit0=19  base0=LSB of Input  dbl_ovrd=off  block_type=slice
      settings: infoedit="Extracts a given range of bits from each input sample and presents it at the output.  The output type is ordinarily unsigned with binary point at zero, but can be Boolean when the slice is one bit wide.<P><P>Hardware notes: In hardware this block costs nothing."  block_version=10.1.3
  Terminator : Terminator
  bram_ctrl : Subsystem
  calc_adc_sum_sq0 : Subsystem
  calc_adc_sum_sq1 : Subsystem
  calc_adc_sum_sq10 : Subsystem
  calc_adc_sum_sq11 : Subsystem
  calc_adc_sum_sq12 : Subsystem
  calc_adc_sum_sq13 : Subsystem
  calc_adc_sum_sq14 : Subsystem
  calc_adc_sum_sq15 : Subsystem
  calc_adc_sum_sq16 : Subsystem
  calc_adc_sum_sq17 : Subsystem
  calc_adc_sum_sq18 : Subsystem
  calc_adc_sum_sq19 : Subsystem
  calc_adc_sum_sq2 : Subsystem
  calc_adc_sum_sq20 : Subsystem
  calc_adc_sum_sq21 : Subsystem
  calc_adc_sum_sq22 : Subsystem
  calc_adc_sum_sq23 : Subsystem
  calc_adc_sum_sq24 : Subsystem
  calc_adc_sum_sq25 : Subsystem
  calc_adc_sum_sq26 : Subsystem
  calc_adc_sum_sq27 : Subsystem
  calc_adc_sum_sq28 : Subsystem
  calc_adc_sum_sq29 : Subsystem
  calc_adc_sum_sq3 : Subsystem
  calc_adc_sum_sq30 : Subsystem
  calc_adc_sum_sq31 : Subsystem
  calc_adc_sum_sq4 : Subsystem
  calc_adc_sum_sq5 : Subsystem
  calc_adc_sum_sq6 : Subsystem
  calc_adc_sum_sq7 : Subsystem
  calc_adc_sum_sq8 : Subsystem
  calc_adc_sum_sq9 : Subsystem
  clip_detect : Subsystem
  edge_detect : casper_library_misc/edge_detect  edge=Rising  polarity=Active High  x_in=[0 0.08 0.08 0.16 0.16 0.24 0.24 0.32 0.32 0.4]  y_in="[-1 -1  1  1  1  1  1  1  1  1]"  x_out=[0.6 0.68 0.68 0.76 0.76 0.84 0.84 0.92 0.92 1]  y_out="[-1 -1  1  1 -1 -1 -1 -1 -1 -1]"
  enable : xps_library/software register  Tag=xps:sw_reg  io_dir=From Processor  arith_type=Unsigned  bitwidth=32  bin_pt=0  sample_period=1  gw_name=roach2_fengine_input_rms_enable_user_data_out
  levels : Subsystem  Tag=xps:bram  AncestorBlock=xps_library/Shared BRAM  mask{arith_type=Unsigned, addr_width=10, data_width=32, reg_prim_output=off, reg_core_output=off, optimization=Minimum_Area, data_bin_pt=0, init_vals=[0:2^10-1], sample_rate=1}
      mask prompts: arith_type "Output Data Type"; addr_width "Address width"; data_width "Data Width"; reg_prim_output "Register Primitive Output"; reg_core_output "Register Core Output"; data_bin_pt "Data Binary Point"; init_vals "Initial 32-bit values (simulation only)"; sample_rate "Sample rate"
      mask init:
      | shared_bram_mask;
      mask TypeOptions arith_type: Unsigned | Signed  (2's comp)
      mask TypeOptions data_width: 8 | 16 | 32 | 64 | 128
      mask TypeOptions optimization: Minimum_Area | Low_Power
  lsb : xbsIndex_r4/Slice  nbits=0  boolean_output=on  mode=Lower Bit Location + Width  bit1=0  base1=MSB of Input  bit0=0  base0=LSB of Input  dbl_ovrd=off  block_type=slice
      settings: block_version=10.1.3
  tag scope: adc_rms_rst=global
  wires:
  Counter/1 -> Slice16/1
  Slice16/1 -> edge_detect/1
  edge_detect/1 -> [adc_rms_rst]
  in/1 -> Bus Selector/1
  Bus Selector/1 -> calc_adc_sum_sq0/1(in)  "<in0>"
  Bus Selector/2 -> calc_adc_sum_sq1/1(in)  "<in1>"
  Bus Selector/3 -> calc_adc_sum_sq2/1(in)  "<in2>"
  Bus Selector/4 -> calc_adc_sum_sq3/1(in)  "<in3>"
  Bus Selector/5 -> calc_adc_sum_sq4/1(in)  "<in4>"
  Bus Selector/6 -> calc_adc_sum_sq5/1(in)  "<in5>"
  Bus Selector/7 -> calc_adc_sum_sq6/1(in)  "<in6>"
  Bus Selector/8 -> calc_adc_sum_sq7/1(in)  "<in7>"
  Bus Selector/9 -> calc_adc_sum_sq8/1(in)  "<in8>"
  Bus Selector/10 -> calc_adc_sum_sq9/1(in)  "<in9>"
  Bus Selector/11 -> calc_adc_sum_sq10/1(in)  "<in10>"
  Bus Selector/12 -> calc_adc_sum_sq11/1(in)  "<in11>"
  Bus Selector/13 -> calc_adc_sum_sq12/1(in)  "<in12>"
  Bus Selector/14 -> calc_adc_sum_sq13/1(in)  "<in13>"
  Bus Selector/15 -> calc_adc_sum_sq14/1(in)  "<in14>"
  Bus Selector/16 -> calc_adc_sum_sq15/1(in)  "<in15>"
  Bus Selector/17 -> calc_adc_sum_sq16/1(in)  "<in16>"
  Bus Selector/18 -> calc_adc_sum_sq17/1(in)  "<in17>"
  Bus Selector/19 -> calc_adc_sum_sq18/1(in)  "<in18>"
  Bus Selector/20 -> calc_adc_sum_sq19/1(in)  "<in19>"
  Bus Selector/21 -> calc_adc_sum_sq20/1(in)  "<in20>"
  Bus Selector/22 -> calc_adc_sum_sq21/1(in)  "<in21>"
  Bus Selector/23 -> calc_adc_sum_sq22/1(in)  "<in22>"
  Bus Selector/24 -> calc_adc_sum_sq23/1(in)  "<in23>"
  Bus Selector/25 -> calc_adc_sum_sq24/1(in)  "<in24>"
  Bus Selector/26 -> calc_adc_sum_sq25/1(in)  "<in25>"
  Bus Selector/27 -> calc_adc_sum_sq26/1(in)  "<in26>"
  Bus Selector/28 -> calc_adc_sum_sq27/1(in)  "<in27>"
  Bus Selector/29 -> calc_adc_sum_sq28/1(in)  "<in28>"
  Bus Selector/30 -> calc_adc_sum_sq29/1(in)  "<in29>"
  Bus Selector/31 -> calc_adc_sum_sq30/1(in)  "<in30>"
  Bus Selector/32 -> calc_adc_sum_sq31/1(in)  "<in31>"
  enable/1 -> lsb/1
  calc_adc_sum_sq0/1(sum_sumsq) -> bram_ctrl/1(adc0)
  calc_adc_sum_sq1/1(sum_sumsq) -> bram_ctrl/2(adc1)
  calc_adc_sum_sq2/1(sum_sumsq) -> bram_ctrl/3(adc2)
  calc_adc_sum_sq3/1(sum_sumsq) -> bram_ctrl/4(adc3)
  calc_adc_sum_sq4/1(sum_sumsq) -> bram_ctrl/5(adc4)
  calc_adc_sum_sq5/1(sum_sumsq) -> bram_ctrl/6(adc5)
  calc_adc_sum_sq6/1(sum_sumsq) -> bram_ctrl/7(adc6)
  calc_adc_sum_sq7/1(sum_sumsq) -> bram_ctrl/8(adc7)
  calc_adc_sum_sq8/1(sum_sumsq) -> bram_ctrl/9(adc8)
  calc_adc_sum_sq9/1(sum_sumsq) -> bram_ctrl/10(adc9)
  calc_adc_sum_sq10/1(sum_sumsq) -> bram_ctrl/11(adc10)
  calc_adc_sum_sq11/1(sum_sumsq) -> bram_ctrl/12(adc11)
  calc_adc_sum_sq12/1(sum_sumsq) -> bram_ctrl/13(adc12)
  calc_adc_sum_sq13/1(sum_sumsq) -> bram_ctrl/14(adc13)
  calc_adc_sum_sq14/1(sum_sumsq) -> bram_ctrl/15(adc14)
  calc_adc_sum_sq15/1(sum_sumsq) -> bram_ctrl/16(adc15)
  calc_adc_sum_sq16/1(sum_sumsq) -> bram_ctrl/17(adc16)
  calc_adc_sum_sq17/1(sum_sumsq) -> bram_ctrl/18(adc17)
  calc_adc_sum_sq18/1(sum_sumsq) -> bram_ctrl/19(adc18)
  calc_adc_sum_sq19/1(sum_sumsq) -> bram_ctrl/20(adc19)
  calc_adc_sum_sq20/1(sum_sumsq) -> bram_ctrl/21(adc20)
  calc_adc_sum_sq21/1(sum_sumsq) -> bram_ctrl/22(adc21)
  calc_adc_sum_sq22/1(sum_sumsq) -> bram_ctrl/23(adc22)
  calc_adc_sum_sq23/1(sum_sumsq) -> bram_ctrl/24(adc23)
  calc_adc_sum_sq24/1(sum_sumsq) -> bram_ctrl/25(adc24)
  calc_adc_sum_sq25/1(sum_sumsq) -> bram_ctrl/26(adc25)
  calc_adc_sum_sq26/1(sum_sumsq) -> bram_ctrl/27(adc26)
  calc_adc_sum_sq27/1(sum_sumsq) -> bram_ctrl/28(adc27)
  calc_adc_sum_sq28/1(sum_sumsq) -> bram_ctrl/29(adc28)
  calc_adc_sum_sq29/1(sum_sumsq) -> bram_ctrl/30(adc29)
  calc_adc_sum_sq30/1(sum_sumsq) -> bram_ctrl/31(adc30)
  calc_adc_sum_sq31/1(sum_sumsq) -> bram_ctrl/32(adc31)
  bram_ctrl/1(addr) -> levels/1(addr)
  bram_ctrl/2(data) -> levels/2(data_in)
  bram_ctrl/3(we) -> levels/3(we)
  lsb/1 -> bram_ctrl/33(en)
  Constant/1 -> enable/1
  levels/1(data_out) -> Terminator/1

== system roach2_fengine/monitor/gpio ==
  sel : Inport
  Constant : xbsIndex_r4/Constant  const=0  gui_display_data_type=Boolean  arith_type=Boolean  n_bits=16  bin_pt=14  preci_type=Single  exp_width=8  frac_width=24  explicit_period=on  period=1  equ=P=C  opselect=C  inp2=PCIN>>17  opr=+  inp1=P  carry=CIN  dbl_ovrd=off  block_type=constant
  Mux : xbsIndex_r4/Mux  inputs=5  en=off  latency=1  precision=Full  arith_type=Unsigned  n_bits=16  bin_pt=14  quantization=Truncate  overflow=Wrap  dbl_ovrd=off  block_type=mux
  Terminator : Terminator
  sync_out_gpio : xps_library/gpio  Tag=xps:gpio  io_group=ROACH2:sync_out  io_dir=out  arith_type=Boolean  bitwidth=1  bin_pt=0  bit_index=0  sample_period=1  use_single_ended=off  use_ddr=off  reg_iob=on  reg_clk_phase=0  termination=None
  wires:
  Mux/1 -> sync_out_gpio/1
  [sync_gpio_in] -> Mux/3
  [sync_in] -> Mux/4
  [eq_clip] -> Mux/6
  sel/1 -> Mux/1
  Constant/1 -> Mux/2
  [adc_clip] -> Mux/5
  sync_out_gpio/1 -> Terminator/1

== system roach2_fengine/monitor/led ==
  sel : Inport
  Mux : xbsIndex_r4/Mux  inputs=2  en=off  latency=1  precision=Full  arith_type=Unsigned  n_bits=16  bin_pt=14  quantization=Truncate  overflow=Wrap  dbl_ovrd=off  block_type=mux
  Mux1 : xbsIndex_r4/Mux  inputs=2  en=off  latency=1  precision=Full  arith_type=Unsigned  n_bits=16  bin_pt=14  quantization=Truncate  overflow=Wrap  dbl_ovrd=off  block_type=mux
  Terminator : Terminator
  Terminator1 : Terminator
  led0 : xps_library/gpio  Tag=xps:gpio  io_group=ROACH2:led  io_dir=out  arith_type=Boolean  bitwidth=1  bin_pt=0  bit_index=0  sample_period=1  use_single_ended=off  use_ddr=off  reg_iob=on  reg_clk_phase=0  termination=None
  led1 : xps_library/gpio  Tag=xps:gpio  io_group=ROACH2:led  io_dir=out  arith_type=Boolean  bitwidth=1  bin_pt=0  bit_index=1  sample_period=1  use_single_ended=off  use_ddr=off  reg_iob=on  reg_clk_phase=0  termination=None
  pulse_ext : Subsystem  mask{pulse_len=2^18}
      mask prompts: pulse_len "Length of Pulse"
      mask init:
      | bits = ceil(log2(pulse_len+1));
  pulse_ext1 : Subsystem  mask{pulse_len=2^23}
      mask prompts: pulse_len "Length of Pulse"
      mask init:
      | bits = ceil(log2(pulse_len+1));
  pulse_ext2 : Subsystem  mask{pulse_len=2^18}
      mask prompts: pulse_len "Length of Pulse"
      mask init:
      | bits = ceil(log2(pulse_len+1));
  pulse_ext3 : Subsystem  mask{pulse_len=2^23}
      mask prompts: pulse_len "Length of Pulse"
      mask init:
      | bits = ceil(log2(pulse_len+1));
  wires:
  led1/1 -> Terminator/1
  sel/1 -> Mux/1, Mux1/1
  [sync_gpio_in] -> pulse_ext1/1(in)
  Mux/1 -> led1/1
  [adc_clip] -> pulse_ext/1(in)
  led0/1 -> Terminator1/1
  Mux1/1 -> led0/1
  [eq_clip] -> pulse_ext2/1(in)
  [sync_in] -> pulse_ext3/1(in)
  pulse_ext2/1(out) -> Mux1/2
  pulse_ext/1(out) -> Mux/2
  pulse_ext1/1(out) -> Mux/3
  pulse_ext3/1(out) -> Mux1/3

== system roach2_fengine/noise/noise0 ==
  seed : Inport
  ld : Inport  Port=2
  Reinterpret : xbsIndex_r4/Reinterpret  force_arith_type=off  arith_type=Unsigned  force_bin_pt=on  bin_pt=7  block_type=reinterpret
      settings: infoedit="Changes signal type without altering the binary representation.   You can changed the signal between signed and unsigned, and relocate the binary point.<P><P>Hardware notes: In hardware this block costs nothing.<P><P>Example:  Suppose the input is 6 bits wide, signed, with 2 fractional bits, and the output is forced to unsigned with 0 fractional bits.  Then an input of -2.0 (1110.00 in binary 2's complement) becomes an output of 56 (111000 in binary)."  block_version=10.1.3
  Reinterpret1 : xbsIndex_r4/Reinterpret  force_arith_type=off  arith_type=Unsigned  force_bin_pt=on  bin_pt=7  block_type=reinterpret
      settings: infoedit="Changes signal type without altering the binary representation.   You can changed the signal between signed and unsigned, and relocate the binary point.<P><P>Hardware notes: In hardware this block costs nothing.<P><P>Example:  Suppose the input is 6 bits wide, signed, with 2 fractional bits, and the output is forced to unsigned with 0 fractional bits.  Then an input of -2.0 (1110.00 in binary 2's complement) becomes an output of 56 (111000 in binary)."  block_version=10.1.3
  Scale : xbsIndex_r4/Scale  scale_factor=4  dbl_ovrd=off  block_type=scale
      settings: infoedit=Scales input by a power of two by adjusting the binary point position.<P><P>Hardware notes: In hardware this block costs nothing.  block_version=10.1.3
  Scale1 : xbsIndex_r4/Scale  scale_factor=4  dbl_ovrd=off  block_type=scale
      settings: infoedit=Scales input by a power of two by adjusting the binary point position.<P><P>Hardware notes: In hardware this block costs nothing.  block_version=10.1.3
  convert : Subsystem  AncestorBlock=casper_library_misc/convert  mask(convert){bin_pt_in=10, n_bits_out=8, bin_pt_out=0, quantization=Round  (unbiased: +/- Inf), overflow=Wrap, latency=1}
      mask prompts: bin_pt_in "Binary Point In"; n_bits_out "Bit Width Out"; bin_pt_out "Binary Point Out"
      mask help:
      | Convert input value to the specified bit width and precision.
      |
      | Does not support upscaling number of fractional bits (eg 8_5 to 8_4 is ok, but not 8_4 to 8_5).
      mask init:
      | convert_init(gcb, ...
      |     'bin_pt_in',bin_pt_in, ...
      |     'n_bits_out',n_bits_out,...
      |     'bin_pt_out',bin_pt_out, ...
      |     'quantization',quantization,...
      |     'overflow',overflow,...
      |     'latency',latency);
      settings: AttributesFormatString=|
      | N_10 ==> 8_0
      | round inf, wrap
      | latency = 1
      mask Help:
      | <pre>
      | Description::
      | 
      | Outputs converted version of the input.
      | 
      | Mask Parameters::
      | 
      | Binary Point In (bin_pt_in): Specifies the binary point location in the input.
      | 
      | Bit Width Out (n_bits_out): Specifies the width of the output.
      | 
      | Binary Point Out (bin_pt_out): Specifies the binary point location in the output.
      | 
      | Quantization (quantization): Specifies quantization handling.
      | 
      | Overflow (overflow): Specifies overflow handling.
      | 
      | Latency (latency): Specifies the latency of the underlying adder block, which is the overall
      | latency of this block as well.
      | </pre>
      mask TypeOptions quantization: Truncate | Round  (unbiased: +/- Inf) | Round  (unbiased: Even Values) | Round  (unbiased: Zero) | Round  (unbiased: Odd Values) | Round  (biased: Up) | Round  (biased: Down)
      mask TypeOptions overflow: Wrap | Saturate | Error
  convert1 : Subsystem  AncestorBlock=casper_library_misc/convert  mask(convert){bin_pt_in=10, n_bits_out=8, bin_pt_out=0, quantization=Round  (unbiased: +/- Inf), overflow=Wrap, latency=1}
      mask prompts: bin_pt_in "Binary Point In"; n_bits_out "Bit Width Out"; bin_pt_out "Binary Point Out"
      mask help:
      | Convert input value to the specified bit width and precision.
      |
      | Does not support upscaling number of fractional bits (eg 8_5 to 8_4 is ok, but not 8_4 to 8_5).
      mask init: same as roach2_fengine/noise/noise0/convert
      settings: AttributesFormatString=|
      | N_10 ==> 8_0
      | round inf, wrap
      | latency = 1
      mask Help:
      | <pre>
      | Description::
      | 
      | Outputs converted version of the input.
      | 
      | Mask Parameters::
      | 
      | Binary Point In (bin_pt_in): Specifies the binary point location in the input.
      | 
      | Bit Width Out (n_bits_out): Specifies the width of the output.
      | 
      | Binary Point Out (bin_pt_out): Specifies the binary point location in the output.
      | 
      | Quantization (quantization): Specifies quantization handling.
      | 
      | Overflow (overflow): Specifies overflow handling.
      | 
      | Latency (latency): Specifies the latency of the underlying adder block, which is the overall
      | latency of this block as well.
      | </pre>
      mask TypeOptions quantization: Truncate | Round  (unbiased: +/- Inf) | Round  (unbiased: Even Values) | Round  (unbiased: Zero) | Round  (unbiased: Odd Values) | Round  (biased: Up) | Round  (biased: Down)
      mask TypeOptions overflow: Wrap | Saturate | Error
  wgn : casper_library_sources/White Gaussian Noise  seed=hex2dec('12345678')
      link override u2n/log_lut: depth=2^11  initVector=sqrt(-2*log(linspace(2^-11,1,2^11)))  distributed_mem=Block RAM  rst=off  init_reg=0  en=off  latency=6  gui_display_data_type=Fixed-point  arith_type=Unsigned  n_bits=9  bin_pt=7  preci_type=Single  exp_width=8  frac_width=24  dbl_ovrd=off  optimize=Area  use_rpm=off
      link override u2n/log_lut: xl_area=[0 0 1 0 0 0 0]
  n1 : Outport
  n2 : Outport  Port=2
  wires:
  Reinterpret1/1 -> n2/1
  Reinterpret/1 -> n1/1
  Scale1/1 -> convert1/1(in)
  Scale/1 -> convert/1(in)
  ld/1 -> wgn/2
  seed/1 -> wgn/1
  convert1/1(out) -> Reinterpret1/1
  convert/1(out) -> Reinterpret/1
  wgn/2 -> Scale1/1
  wgn/1 -> Scale/1
